simulink model "TosAeb"  (R2021a)
settings: Solver=FixedStepDiscrete  StartTime=0.0  StopTime=inf  FixedStep=0.01  RelTol=1e-3
undefined symbols (not defined in the file or in its folder): ACCModSts, AccSts1, AEB_ObjectMovingOrientation, AEB_ObjectMovingState, CN_BTCActive, CN_CurrentGearR, CN_CurrentGearR_DHT, CN_PTCActive, CN_VDCActive, CN_VSC_EspOff, CN_VSC_FCW_AEB_Error, CN_VSC_FCW_AEB_NoDispay, CN_VSC_FCW_AEB_PerfmDgdtn, CN_VSC_FCW_AEB_TempUnAvlbl, CN_VSC_VehStandstill, CNaccelAvoidMax, CNaccelAvoidMin, CNbrakePedalPress, CNcomfortStrLeftAccelMax, CNcomfortStrLeftAccelMin, CNcomfortStrRightAccelMax, CNcomfortStrRightAccelMin, CNDrvDoorOpen, CNDrvModeEco, CNDrvModeNorm, CNDrvModeOther, CNDrvModeSport, CNDrvSBRreminder, CNNull, CNobjInvalidID, CNPassDoorOpen, CNPassSBRreminder, CNPEBSAccelNegInf, CNPEBSAccelNull, CNPEBSTimeInf, CNPEBSTimeNull, CNSTOPMAX, CNSTOPNULL, CNsubjWidth, CNSwtOFF, CNSwtON, CNTTCMAX, CNTTCNULL, CNVehConfigAEBCycNone, CNVehConfigAEBNone, CNVehConfigAEBPedNone, CNVehConfigFCWNone, CNVehConfigJANone, CollisionSeverity, FALSE, FCWSnvty, JACycObjCalcInfoDefaultParams, JAPedObjCalcInfoDefaultParams, JAVehObjCalcInfoDefaultParams, latCycObjCalcInfoDefaultParams, latPedObjCalcInfoDefaultParams, longObjCalcInfoDefaultParams, ModSts, movingStatus, ObjectType ... (+1112)
libraries: simulink x3031, AEBSFunctionLib x50, Ta_Library x33, AEBSStateMachineLib x23, Mylibrary x12, simulink_extras x7, ObjSelect_lib x3, dspstat3 x2
(part 74 of 102)

== system TosAeb/FcwAebJa_Step_sys/ALG_AEBSystem/ALG_AEBController/AEB_LongTgtInfoCalc/LongObjCalcInfoCalc/AEBS_LongCollisionAssesApp/collisionSeverityHighCalc/collisionSeverityHighCalc2/collisionSeverityHighPhase3/Phase3TimeCalc/tMaxReduceCalc ==
  note: if subjAccelWorkPhase2 < 0
      | 	Calculate tMaxReduce;
      | else
      | 	tMaxReduce = +inf;
  subjLongAccelWorkPhase2 : Inport
  velMaxReudce : Inport  Port=2
  subjVelWorkPhase2 : Inport  Port=3
  If : If  IfExpression=u1 < 0
  Merge : Merge
  TimeMaxReduceCalc : Subsystem  TreatAsAtomicUnit=on
  TimeMaxReduceInf : Subsystem  TreatAsAtomicUnit=on
  tMaxReduce : Outport
  wires:
  If/1 -> TimeMaxReduceCalc/Action
  TimeMaxReduceInf/1(tMaxReduce) -> Merge/2
  TimeMaxReduceCalc/1(tMaxReduce) -> Merge/1
  subjLongAccelWorkPhase2/1 -> If/1, TimeMaxReduceCalc/1(subjLongAccelWorkPhase2)
  velMaxReudce/1 -> TimeMaxReduceCalc/2(velMaxReudce)
  subjVelWorkPhase2/1 -> TimeMaxReduceCalc/3(subjVelWorkPhase2)
  If/2 -> [TIMEINF]
  [TIMEINF] -> TimeMaxReduceInf/Action  "timeMaxReduceInf"
  Merge/1 -> tMaxReduce/1  "tMaxReduce"

== system TosAeb/FcwAebJa_Step_sys/ALG_AEBSystem/ALG_AEBController/AEB_LongTgtInfoCalc/LongObjCalcInfoCalc/AEBS_LongCollisionAssesApp/collisionSeverityHighCalc/collisionSeverityHighCalc2/collisionSeverityHighPhase3/Phase3TimeCalc/tObjStopCalc ==
  accelAbs : Inport
  velAbs : Inport  Port=2
  StopTimeFlow : Stateflow chart  TreatAsAtomicUnit=on
  tStop : Outport
  wires:
  velAbs/1 -> StopTimeFlow/2(velRel)  "velAbs"
  accelAbs/1 -> StopTimeFlow/1(accelRel)  "accelAbs"
  StopTimeFlow/1(tStop) -> tStop/1  "tStop"

== system TosAeb/AEB_Objsel/TA_Processor/AEB_ObjSelect/TargetInfoCheck/Data_precess/TA_EffectCalculate/Obj_FilterFlgCalculate/JA_ObjFilter/CollisionProbCalc/Subsystem/ObjBoundingBox/ObjLLCoordinate ==
  inputSignals : Inport
  Add : Sum  Inputs=+-  OutDataTypeStr=single
  Add1 : Sum  OutDataTypeStr=single
  Bus Selector : BusSelector  OutputSignals=dxPreRefPointObj,ObjWidthCorr,sincosCoef.sinCoef
  Bus Selector1 : BusSelector  OutputSignals=dyPreRefPointObj,ObjWidthCorr,sincosCoef.cosCoef
  Gain : Gain  Gain=0.5  OutDataTypeStr=single
  Gain1 : Gain  Gain=0.5  OutDataTypeStr=single
  Product1 : Product  OutDataTypeStr=single
  Product3 : Product  OutDataTypeStr=single
  dxPre_LLObj : Outport
  dyPreLLObj : Outport  Port=2
  wires:
  Bus Selector1/1 -> Add1/1  "<dyPreRefPointObj>"
  Bus Selector1/3 -> Product3/2  "<cosCoef>"
  Bus Selector1/2 -> Product3/1  "<ObjWidthCorr>"
  Product3/1 -> Gain/1
  Add1/1 -> dyPreLLObj/1
  Add/1 -> dxPre_LLObj/1
  Bus Selector/1 -> Add/1  "<dxPreRefPointObj>"
  Product1/1 -> Gain1/1
  Bus Selector/3 -> Product1/2  "<sinCoef>"
  Bus Selector/2 -> Product1/1  "<ObjWidthCorr>"
  inputSignals/1 -> Bus Selector1/1, Bus Selector/1
  Gain/1 -> Add1/2
  Gain1/1 -> Add/2

== system TosAeb/AEB_Objsel/TA_Processor/AEB_ObjSelect/TargetInfoCheck/Data_precess/TA_EffectCalculate/Obj_FilterFlgCalculate/JA_ObjFilter/CollisionProbCalc/Subsystem/ObjBoundingBox/ObjLUCoordinate ==
  inputSignals : Inport
  Add : Sum  Inputs=++-  OutDataTypeStr=single
  Add1 : Sum  Inputs=+++  OutDataTypeStr=single
  Bus Selector : BusSelector  OutputSignals=dxPreRefPointObj,ObjLengthCorr,sincosCoef.cosCoef,ObjWidthCorr,sincosCoef.sinCoef
  Bus Selector1 : BusSelector  OutputSignals=dyPreRefPointObj,ObjLengthCorr,sincosCoef.sinCoef,ObjWidthCorr,sincosCoef.cosCoef
  Gain : Gain  Gain=0.5  OutDataTypeStr=single
  Gain1 : Gain  Gain=0.5  OutDataTypeStr=single
  Product{,1..3} (4 alike) : Product  OutDataTypeStr=single
  dxPreLUObj : Outport
  dyPreLUobj : Outport  Port=2
  wires:
  inputSignals/1 -> Bus Selector1/1, Bus Selector/1
  Bus Selector/2 -> Product/1  "<ObjLengthCorr>"
  Bus Selector/3 -> Product/2  "<cosCoef>"
  Bus Selector/4 -> Product1/1  "<ObjWidthCorr>"
  Bus Selector/5 -> Product1/2  "<sinCoef>"
  Product1/1 -> Gain1/1
  Bus Selector/1 -> Add/1  "<dxPreRefPointObj>"
  Product/1 -> Add/2
  Add/1 -> dxPreLUObj/1
  Add1/1 -> dyPreLUobj/1
  Product2/1 -> Add1/2
  Product3/1 -> Gain/1
  Bus Selector1/2 -> Product2/1  "<ObjLengthCorr>"
  Bus Selector1/4 -> Product3/1  "<ObjWidthCorr>"
  Bus Selector1/3 -> Product2/2  "<sinCoef>"
  Bus Selector1/5 -> Product3/2  "<cosCoef>"
  Bus Selector1/1 -> Add1/1  "<dyPreRefPointObj>"
  Gain/1 -> Add1/3
  Gain1/1 -> Add/3

== system TosAeb/AEB_Objsel/TA_Processor/AEB_ObjSelect/TargetInfoCheck/Data_precess/TA_EffectCalculate/Obj_FilterFlgCalculate/JA_ObjFilter/CollisionProbCalc/Subsystem/ObjBoundingBox/ObjRLCoordinate ==
  inputSignals : Inport
  Add : Sum  OutDataTypeStr=single
  Add1 : Sum  Inputs=+-  OutDataTypeStr=single
  Bus Selector : BusSelector  OutputSignals=dxPreRefPointObj,ObjWidthCorr,sincosCoef.sinCoef
  Bus Selector1 : BusSelector  OutputSignals=dyPreRefPointObj,ObjWidthCorr,sincosCoef.cosCoef
  Gain : Gain  Gain=0.5  OutDataTypeStr=single
  Gain1 : Gain  Gain=0.5  OutDataTypeStr=single
  Product1 : Product  OutDataTypeStr=single
  Product3 : Product  OutDataTypeStr=single
  dxPreRLObj : Outport
  dyPreRLObj : Outport  Port=2
  wires:
  Bus Selector1/1 -> Add1/1  "<dyPreRefPointObj>"
  Bus Selector1/3 -> Product3/2  "<cosCoef>"
  Bus Selector1/2 -> Product3/1  "<ObjWidthCorr>"
  Product3/1 -> Gain1/1
  Add1/1 -> dyPreRLObj/1
  Add/1 -> dxPreRLObj/1
  Bus Selector/1 -> Add/1  "<dxPreRefPointObj>"
  Product1/1 -> Gain/1
  Bus Selector/3 -> Product1/2  "<sinCoef>"
  Bus Selector/2 -> Product1/1  "<ObjWidthCorr>"
  inputSignals/1 -> Bus Selector1/1, Bus Selector/1
  Gain/1 -> Add/2
  Gain1/1 -> Add1/2

== system TosAeb/AEB_Objsel/TA_Processor/AEB_ObjSelect/TargetInfoCheck/Data_precess/TA_EffectCalculate/Obj_FilterFlgCalculate/JA_ObjFilter/CollisionProbCalc/Subsystem/ObjBoundingBox/ObjRUCoordinate ==
  inputSignals : Inport
  Add : Sum  Inputs=+++  OutDataTypeStr=single
  Add1 : Sum  Inputs=++-  OutDataTypeStr=single
  Bus Selector : BusSelector  OutputSignals=dxPreRefPointObj,ObjLengthCorr,sincosCoef.cosCoef,ObjWidthCorr,sincosCoef.sinCoef
  Bus Selector1 : BusSelector  OutputSignals=dyPreRefPointObj,ObjLengthCorr,sincosCoef.sinCoef,ObjWidthCorr,sincosCoef.cosCoef
  Gain : Gain  Gain=0.5  OutDataTypeStr=single
  Gain1 : Gain  Gain=0.5  OutDataTypeStr=single
  Product{,1..3} (4 alike) : Product  OutDataTypeStr=single
  dxPreRUObj : Outport
  dyPreRUObj : Outport  Port=2
  wires:
  inputSignals/1 -> Bus Selector1/1, Bus Selector/1
  Bus Selector/2 -> Product/1  "<ObjLengthCorr>"
  Bus Selector/3 -> Product/2  "<cosCoef>"
  Bus Selector/4 -> Product1/1  "<ObjWidthCorr>"
  Bus Selector/5 -> Product1/2  "<sinCoef>"
  Product1/1 -> Gain1/1
  Bus Selector/1 -> Add/1  "<dxPreRefPointObj>"
  Product/1 -> Add/2
  Add/1 -> dxPreRUObj/1
  Add1/1 -> dyPreRUObj/1
  Product2/1 -> Add1/2
  Product3/1 -> Gain/1
  Bus Selector1/2 -> Product2/1  "<ObjLengthCorr>"
  Bus Selector1/4 -> Product3/1  "<ObjWidthCorr>"
  Bus Selector1/3 -> Product2/2  "<sinCoef>"
  Bus Selector1/5 -> Product3/2  "<cosCoef>"
  Bus Selector1/1 -> Add1/1  "<dyPreRefPointObj>"
  Gain/1 -> Add1/3
  Gain1/1 -> Add/3

== system TosAeb/AEB_Objsel/TA_Processor/AEB_ObjSelect/TargetInfoCheck/Data_precess/TA_EffectCalculate/Obj_FilterFlgCalculate/JA_ObjFilter/CollisionProbCalc/Subsystem/ObjBoundingBoxRebuild/Subsystem ==
  SubDxFR : Inport
  SubDxFL : Inport  Port=2
  SubDxRL : Inport  Port=3
  SubDxRR : Inport  Port=4
  Add{,1..3} (4 alike) : Sum  Inputs=+-
  Gain : Gain  Gain=1/3
  Gain1 : Gain  Gain=1/3
  Out2 : Outport
  Out1 : Outport  Port=2
  wires:
  SubDxFR/1 -> Add1/1, Add/1
  SubDxRR/1 -> Add/2
  Add/1 -> Gain/1
  Gain/1 -> Add1/2
  Add1/1 -> Out1/1
  Add2/1 -> Gain1/1
  Gain1/1 -> Add3/2
  Add3/1 -> Out2/1
  SubDxRL/1 -> Add2/2
  SubDxFL/1 -> Add3/1, Add2/1

== system TosAeb/AEB_Objsel/TA_Processor/AEB_ObjSelect/TargetInfoCheck/Data_precess/TA_EffectCalculate/Obj_FilterFlgCalculate/JA_ObjFilter/CollisionProbCalc/Subsystem/ObjBoundingBoxRebuild/Subsystem1 ==
  SubDyFR : Inport
  SubDyFL : Inport  Port=2
  SubDyRL : Inport  Port=3
  SubDyRR : Inport  Port=4
  Add{,1..3} (4 alike) : Sum  Inputs=+-
  Gain : Gain  Gain=1/3
  Gain1 : Gain  Gain=1/3
  Out2 : Outport
  Out1 : Outport  Port=2
  wires:
  SubDyFL/1 -> Add2/1, Add3/1
  SubDyRL/1 -> Add2/2
  Add3/1 -> Out2/1
  Gain1/1 -> Add3/2
  Add2/1 -> Gain1/1
  Add1/1 -> Out1/1
  Gain/1 -> Add1/2
  Add/1 -> Gain/1
  SubDyRR/1 -> Add/2
  SubDyFR/1 -> Add/1, Add1/1

== system TosAeb/AEB_Objsel/TA_Processor/AEB_ObjSelect/TargetInfoCheck/Data_precess/TA_EffectCalculate/Obj_FilterFlgCalculate/JA_ObjFilter/CollisionProbCalc/Subsystem/ObjBoundingBoxRebuild/Subsystem2 ==
  ObjDxFR : Inport
  ObjDxFL : Inport  Port=2
  ObjDxRL : Inport  Port=3
  ObjDxRR : Inport  Port=4
  Add : Sum  Inputs=+-
  Add1 : Sum
  Add2 : Sum  Inputs=+-
  Add3 : Sum
  Gain : Gain  Gain=1/3
  Gain1 : Gain  Gain=1/3
  Out2 : Outport
  Out1 : Outport  Port=2
  wires:
  Add/1 -> Gain/1
  Add1/1 -> Out1/1
  Add2/1 -> Gain1/1
  Add3/1 -> Out2/1
  ObjDxRL/1 -> Add3/2, Add2/2
  ObjDxFL/1 -> Add2/1
  ObjDxFR/1 -> Add/1
  ObjDxRR/1 -> Add1/2, Add/2
  Gain/1 -> Add1/1
  Gain1/1 -> Add3/1

== system TosAeb/AEB_Objsel/TA_Processor/AEB_ObjSelect/TargetInfoCheck/Data_precess/TA_EffectCalculate/Obj_FilterFlgCalculate/JA_ObjFilter/CollisionProbCalc/Subsystem/ObjBoundingBoxRebuild/Subsystem3 ==
  ObjDyFR : Inport
  ObjDyFL : Inport  Port=2
  ObjDyRL : Inport  Port=3
  ObjDyRR : Inport  Port=4
  Add : Sum  Inputs=+-
  Add1 : Sum
  Add2 : Sum  Inputs=+-
  Add3 : Sum
  Gain : Gain  Gain=1/3
  Gain1 : Gain  Gain=1/3
  Out2 : Outport
  Out1 : Outport  Port=2
  wires:
  ObjDyRR/1 -> Add1/2, Add/2
  ObjDyFR/1 -> Add/1
  ObjDyFL/1 -> Add2/1
  ObjDyRL/1 -> Add3/2, Add2/2
  Add3/1 -> Out2/1
  Add2/1 -> Gain1/1
  Add1/1 -> Out1/1
  Add/1 -> Gain/1
  Gain1/1 -> Add3/1
  Gain/1 -> Add1/1

== system TosAeb/AEB_Objsel/TA_Processor/AEB_ObjSelect/TargetInfoCheck/Data_precess/TA_EffectCalculate/Obj_FilterFlgCalculate/JA_ObjFilter/CollisionProbCalc/Subsystem/PreCrossFlgCalcFlow/VectorCrossAlgorithm/If Action Subsystem ==
  Action Port : ActionPort  ActionPortLabel=if(u1)
  Constant : Constant  Value=0  OutDataTypeStr=boolean
  CrossFlg : Outport
  wires:
  Constant/1 -> CrossFlg/1

== system TosAeb/AEB_Objsel/TA_Processor/AEB_ObjSelect/TargetInfoCheck/Data_precess/TA_EffectCalculate/Obj_FilterFlgCalculate/JA_ObjFilter/CollisionProbCalc/Subsystem/PreCrossFlgCalcFlow/VectorCrossAlgorithm/If Action Subsystem1 ==
  vectorMulit : Inport
  Coordinations : Inport  Port=2
  Action Port : ActionPort  ActionPortLabel=else
  Bus Selector : BusSelector  OutputSignals=m2,m1,y1,n1,n2,x1
  Bus Selector1 : BusSelector  OutputSignals=x2,x1,y1,n1,y2,m1
  Subsystem : Subsystem
  t : Subsystem
  u : Subsystem
  CrossFlg : Outport
  wires:
  Coordinations/1 -> Bus Selector1/1, Bus Selector/1
  Bus Selector/2 -> t/2(m1)  "<m1>"
  Bus Selector/3 -> t/3(y1)  "<y1>"
  Bus Selector/1 -> t/1(m2)  "<m2>"
  Bus Selector/4 -> t/4(n1)  "<n1>"
  Bus Selector/5 -> t/5(n2)  "<n2>"
  Bus Selector/6 -> t/6(x1)  "<x1>"
  Bus Selector1/3 -> u/3(y1)  "<y1>"
  Bus Selector1/2 -> u/2(x1)  "<x1>"
  Bus Selector1/4 -> u/4(n1)  "<n1>"
  Bus Selector1/1 -> u/1(x2)  "<x2>"
  Bus Selector1/5 -> u/5(y2)  "<y2>"
  Bus Selector1/6 -> u/6(m1)  "<m1>"
  t/1(temp_t) -> Subsystem/1(temp_u)
  u/1(temp_u) -> Subsystem/2(temp_t)
  Subsystem/1(CrossFlg) -> CrossFlg/1
  vectorMulit/1 -> Subsystem/3(vectorMulit)

== system TosAeb/AEB_Objsel/TA_Processor/AEB_ObjSelect/TargetInfoCheck/Data_precess/TA_EffectCalculate/Obj_FilterFlgCalculate/JA_ObjFilter/CollisionProbCalc/Subsystem/PreCrossFlgCalcFlow/VectorCrossAlgorithm/VectorMulit ==
  x1 : Inport  OutDataTypeStr=single
  x2 : Inport  Port=2  OutDataTypeStr=single
  n2 : Inport  Port=3  OutDataTypeStr=single
  n1 : Inport  Port=4  OutDataTypeStr=single
  y2 : Inport  Port=5  OutDataTypeStr=single
  y1 : Inport  Port=6  OutDataTypeStr=single
  m2 : Inport  Port=7  OutDataTypeStr=single
  m1 : Inport  Port=8  OutDataTypeStr=single
  Product : Product  OutDataTypeStr=single
  Product1 : Product  OutDataTypeStr=single
  Subtract{1..4} (4 alike) : Sum  Inputs=+-  OutDataTypeStr=single
  Subtract5 : Sum  Inputs=+-
  vectorMulit : Outport
  wires:
  x1/1 -> Subtract2/2
  x2/1 -> Subtract2/1
  Subtract2/1 -> Product/1
  Subtract3/1 -> Product/2
  y2/1 -> Subtract4/1
  y1/1 -> Subtract4/2
  m2/1 -> Subtract1/1
  m1/1 -> Subtract1/2
  Subtract4/1 -> Product1/1
  Subtract1/1 -> Product1/2
  Product/1 -> Subtract5/1
  Product1/1 -> Subtract5/2
  Subtract5/1 -> vectorMulit/1
  n2/1 -> Subtract3/1
  n1/1 -> Subtract3/2

== system TosAeb/AEB_Objsel/TA_Processor/AEB_ObjSelect/TargetInfoCheck/Data_precess/TA_EffectCalculate/Obj_FilterFlgCalculate/JA_ObjFilter/CollisionProbCalc/Subsystem/SubjBoundingBox/SubjLLCoordinate ==
  inputSignals : Inport
  Add2 : Sum  Inputs=+--  OutDataTypeStr=single
  Add3 : Sum  Inputs=+-+  OutDataTypeStr=single
  Bus Selector2 : BusSelector  OutputSignals=dxPreRefPoint,dxRearBumperToRearAxis,sincosCoef.cosCoef,subjWidth,sincosCoef.sinCoef
  Bus Selector3 : BusSelector  OutputSignals=dyPreRefPoint,dxRearBumperToRearAxis,sincosCoef.sinCoef,subjWidth,sincosCoef.cosCoef
  Gain2 : Gain  Gain=0.5  OutDataTypeStr=single
  Gain3 : Gain  Gain=0.5  OutDataTypeStr=single
  Product{4..7} (4 alike) : Product  OutDataTypeStr=single
  dxPreLL : Outport
  dyPreLL : Outport  Port=2
  wires:
  Gain3/1 -> Add3/3
  Bus Selector3/3 -> Product6/2  "<sinCoef>"
  Add3/1 -> dyPreLL/1
  Product7/1 -> Gain3/1
  Product6/1 -> Add3/2
  Product4/1 -> Add2/2
  Bus Selector3/5 -> Product7/2  "<cosCoef>"
  Product5/1 -> Gain2/1
  Gain2/1 -> Add2/3
  Add2/1 -> dxPreLL/1
  Bus Selector3/4 -> Product7/1  "<subjWidth>"
  Bus Selector3/2 -> Product6/1  "<dxRearBumperToRearAxis>"
  Bus Selector3/1 -> Add3/1  "<dyPreRefPoint>"
  inputSignals/1 -> Bus Selector3/1, Bus Selector2/1
  Bus Selector2/4 -> Product5/1  "<subjWidth>"
  Bus Selector2/2 -> Product4/1  "<dxRearBumperToRearAxis>"
  Bus Selector2/3 -> Product4/2  "<cosCoef>"
  Bus Selector2/1 -> Add2/1  "<dxPreRefPoint>"
  Bus Selector2/5 -> Product5/2  "<sinCoef>"

== system TosAeb/AEB_Objsel/TA_Processor/AEB_ObjSelect/TargetInfoCheck/Data_precess/TA_EffectCalculate/Obj_FilterFlgCalculate/JA_ObjFilter/CollisionProbCalc/Subsystem/SubjBoundingBox/SubjLUCoordinate ==
  inputSignals : Inport
  Add : Sum  Inputs=++-  OutDataTypeStr=single
  Add1 : Sum  Inputs=+++  OutDataTypeStr=single
  Bus Selector : BusSelector  OutputSignals=dxPreRefPoint,dxFrontBumperToRearAxis,sincosCoef.cosCoef,subjWidth,sincosCoef.sinCoef
  Bus Selector1 : BusSelector  OutputSignals=dyPreRefPoint,dxFrontBumperToRearAxis,sincosCoef.sinCoef,subjWidth,sincosCoef.cosCoef
  Gain : Gain  Gain=0.5  OutDataTypeStr=single
  Gain1 : Gain  Gain=0.5  OutDataTypeStr=single
  Product{,1..3} (4 alike) : Product  OutDataTypeStr=single
  dxPreLU : Outport
  dyPreLU : Outport  Port=2
  wires:
  Bus Selector/4 -> Product1/1  "<subjWidth>"
  Product3/1 -> Gain1/1
  Add/1 -> dxPreLU/1
  Bus Selector1/1 -> Add1/1  "<dyPreRefPoint>"
  Gain1/1 -> Add1/3
  Bus Selector1/4 -> Product3/1  "<subjWidth>"
  Product2/1 -> Add1/2
  Bus Selector/3 -> Product/2  "<cosCoef>"
  Add1/1 -> dyPreLU/1
  Bus Selector/5 -> Product1/2  "<sinCoef>"
  Bus Selector/2 -> Product/1  "<dxFrontBumperToRearAxis>"
  Bus Selector1/2 -> Product2/1  "<dxFrontBumperToRearAxis>"
  inputSignals/1 -> Bus Selector1/1, Bus Selector/1
  Bus Selector1/5 -> Product3/2  "<cosCoef>"
  Bus Selector/1 -> Add/1  "<dxPreRefPoint>"
  Gain/1 -> Add/3
  Product1/1 -> Gain/1
  Product/1 -> Add/2
  Bus Selector1/3 -> Product2/2  "<sinCoef>"

== system TosAeb/AEB_Objsel/TA_Processor/AEB_ObjSelect/TargetInfoCheck/Data_precess/TA_EffectCalculate/Obj_FilterFlgCalculate/JA_ObjFilter/CollisionProbCalc/Subsystem/SubjBoundingBox/SubjRLCoordinate ==
  inputSignals : Inport
  Add6 : Sum  Inputs=+-+  OutDataTypeStr=single
  Add7 : Sum  Inputs=+--  OutDataTypeStr=single
  Bus Selector6 : BusSelector  OutputSignals=dxPreRefPoint,dxRearBumperToRearAxis,sincosCoef.cosCoef,subjWidth,sincosCoef.sinCoef
  Bus Selector7 : BusSelector  OutputSignals=dyPreRefPoint,dxRearBumperToRearAxis,sincosCoef.sinCoef,subjWidth,sincosCoef.cosCoef
  Gain6 : Gain  Gain=0.5  OutDataTypeStr=single
  Gain7 : Gain  Gain=0.5  OutDataTypeStr=single
  Product{12..15} (4 alike) : Product  OutDataTypeStr=single
  dxPreRL : Outport
  dyPreRL : Outport  Port=2
  wires:
  Product12/1 -> Add6/2
  Gain6/1 -> Add6/3
  Product13/1 -> Gain6/1
  Add6/1 -> dxPreRL/1
  Bus Selector6/4 -> Product13/1  "<subjWidth>"
  Bus Selector6/5 -> Product13/2  "<sinCoef>"
  Bus Selector6/1 -> Add6/1  "<dxPreRefPoint>"
  Bus Selector6/2 -> Product12/1  "<dxRearBumperToRearAxis>"
  Bus Selector6/3 -> Product12/2  "<cosCoef>"
  inputSignals/1 -> Bus Selector7/1, Bus Selector6/1
  Add7/1 -> dyPreRL/1
  Product14/1 -> Add7/2
  Gain7/1 -> Add7/3
  Bus Selector7/1 -> Add7/1  "<dyPreRefPoint>"
  Bus Selector7/2 -> Product14/1  "<dxRearBumperToRearAxis>"
  Bus Selector7/4 -> Product15/1  "<subjWidth>"
  Product15/1 -> Gain7/1
  Bus Selector7/5 -> Product15/2  "<cosCoef>"
  Bus Selector7/3 -> Product14/2  "<sinCoef>"

== system TosAeb/AEB_Objsel/TA_Processor/AEB_ObjSelect/TargetInfoCheck/Data_precess/TA_EffectCalculate/Obj_FilterFlgCalculate/JA_ObjFilter/CollisionProbCalc/Subsystem/SubjBoundingBox/SubjRUCoordinate ==
  inputSignals : Inport
  Add4 : Sum  Inputs=+++  OutDataTypeStr=single
  Add5 : Sum  Inputs=++-  OutDataTypeStr=single
  Bus Selector4 : BusSelector  OutputSignals=dxPreRefPoint,dxFrontBumperToRearAxis,sincosCoef.cosCoef,subjWidth,sincosCoef.sinCoef
  Bus Selector5 : BusSelector  OutputSignals=dyPreRefPoint,dxFrontBumperToRearAxis,sincosCoef.sinCoef,subjWidth,sincosCoef.cosCoef
  Gain4 : Gain  Gain=0.5  OutDataTypeStr=single
  Gain5 : Gain  Gain=0.5  OutDataTypeStr=single
  Product{8..11} (4 alike) : Product  OutDataTypeStr=single
  dxPreRU : Outport
  dyPreRU : Outport  Port=2
  wires:
  Bus Selector4/4 -> Product9/1  "<subjWidth>"
  Bus Selector4/5 -> Product9/2  "<sinCoef>"
  Bus Selector4/3 -> Product8/2  "<cosCoef>"
  Bus Selector4/1 -> Add4/1  "<dxPreRefPoint>"
  inputSignals/1 -> Bus Selector5/1, Bus Selector4/1
  Bus Selector4/2 -> Product8/1  "<dxFrontBumperToRearAxis>"
  Product9/1 -> Gain4/1
  Bus Selector5/4 -> Product11/1  "<subjWidth>"
  Product10/1 -> Add5/2
  Product11/1 -> Gain5/1
  Add5/1 -> dyPreRU/1
  Gain5/1 -> Add5/3
  Bus Selector5/2 -> Product10/1  "<dxFrontBumperToRearAxis>"
  Bus Selector5/3 -> Product10/2  "<sinCoef>"
  Bus Selector5/5 -> Product11/2  "<cosCoef>"
  Add4/1 -> dxPreRU/1
  Gain4/1 -> Add4/3
  Product8/1 -> Add4/2
  Bus Selector5/1 -> Add5/1  "<dyPreRefPoint>"

== system TosAeb/AEB_Objsel/TA_Processor/AEB_ObjSelect/TargetInfoCheck/Data_precess/TA_EffectCalculate/Obj_FilterFlgCalculate/JA_ObjFilter/CollisionProbCalc/Subsystem/Subsystem/CalculateHeading ==
  dx : Inport
  dy : Inport  Port=2
  atan2 : Trigonometry  Operator=atan2
  PredHostHeading : Outport
  wires:
  dx/1 -> atan2/1
  dy/1 -> atan2/2
  atan2/1 -> PredHostHeading/1  "PredHostHeading"

== system TosAeb/AEB_Objsel/TA_Processor/AEB_ObjSelect/TargetInfoCheck/Data_precess/TA_EffectCalculate/Obj_FilterFlgCalculate/JA_ObjFilter/CollisionProbCalc/Subsystem/Subsystem/SegmentComponents ==
  note: 判断预测时间的位置，与第一段时间做差，然后除以第一段与第四段的差值，输出数
      | 值为0到1的数
  note: 驾驶员预测前方道路分为4段，输出为每段的4个点的横纵坐标值，然后做拟合处理
  PahEstimn : Inport
  PredictionTime : Inport  Port=2
  Add : Sum
  Bus Selector : BusSelector  OutputSignals=CtrlAryLgt,CtrlAryLat
  Compare To Constant : simulink/Logic and Bit Operations/Compare To Constant  relop=<=  const=1  OutDataTypeStr=boolean
  Constant : Constant  OutDataTypeStr=single
  LatPosition : MATLAB Function  TreatAsAtomicUnit=on
  LongPosition : MATLAB Function  TreatAsAtomicUnit=on
  Selector : Selector  InputPortWidth=4  IndexOptions=Index vector (dialog)  Indices=1  OutputSizes=1
  Selector1 : Selector  InputPortWidth=4  IndexOptions=Index vector (dialog)  Indices=2  OutputSizes=1
  Selector2 : Selector  InputPortWidth=4  IndexOptions=Index vector (dialog)  Indices=3  OutputSizes=1
  Selector3 : Selector  InputPortWidth=4  IndexOptions=Index vector (dialog)  Indices=4  OutputSizes=1
  Selector4 : Selector  InputPortWidth=4  IndexOptions=Index vector (dialog)  Indices=1  OutputSizes=1
  Selector5 : Selector  InputPortWidth=4  IndexOptions=Index vector (dialog)  Indices=2  OutputSizes=1
  Selector6 : Selector  InputPortWidth=4  IndexOptions=Index vector (dialog)  Indices=3  OutputSizes=1
  Selector7 : Selector  InputPortWidth=4  IndexOptions=Index vector (dialog)  Indices=4  OutputSizes=1
  Switch : Switch  Criteria=u2 ~= 0
  PredHostPosnLgt : Outport
  dx : Outport  Port=2
  PredHostPosnLat : Outport  Port=3
  dy : Outport  Port=4
  wires:
  Bus Selector/2 -> Selector6/1, Selector7/1, Selector4/1, Selector5/1  "<CtrlAryLat>"
  Bus Selector/1 -> Selector2/1, Selector1/1, Selector/1, Selector3/1  "<CtrlAryLgt>"
  PahEstimn/1 -> Bus Selector/1
  Selector1/1 -> LongPosition/2(P1)  "P1x"
  Selector/1 -> LongPosition/1(P0)  "P0x"
  Selector2/1 -> LongPosition/3(P2)  "P2x"
  Selector3/1 -> LongPosition/4(P3)  "P3x"
  Selector4/1 -> LatPosition/1(P0)  "P0y"
  Selector5/1 -> LatPosition/2(P1)  "P1y"
  Selector6/1 -> LatPosition/3(P2)  "P2y"
  Selector7/1 -> LatPosition/4(P3)  "P3y"
  LongPosition/1(x) -> PredHostPosnLgt/1  "PredHostPosnLgt"
  LongPosition/2(dx) -> dx/1
  LatPosition/1(y) -> PredHostPosnLat/1  "PredHostPosnLat"
  LatPosition/2(dy) -> dy/1
  PredictionTime/1 -> Compare To Constant/1, Add/1, Switch/1
  Compare To Constant/1 -> Switch/2
  Constant/1 -> Add/2
  Add/1 -> Switch/3
  Switch/1 -> [tau]
  [tau] -> LatPosition/5(tau)
  [tau] -> LongPosition/5(tau)

== system TosAeb/AEB_Objsel/TA_Processor/AEB_ObjSelect/TargetInfoCheck/Data_precess/TA_EffectCalculate/Obj_FilterFlgCalculate/JA_ObjFilter/CollisionProbCalc/Subsystem/ay_Filt/ay1 ==
  ay : Inport
  Bus Creator : BusCreator  Inputs=2
  Bus Creator1 : BusCreator  Inputs=2
  Compare To Constant : simulink/Logic and Bit Operations/Compare To Constant  relop=>=  const=0.01  OutDataTypeStr=boolean
  Constant : Constant  Value=0  OutDataTypeStr=single
  Constant1 : Constant  Value=0  OutDataTypeStr=single
  Constant2 : Constant  OutDataTypeStr=single
  Switch : Switch  Criteria=u2 ~= 0
  Out_ay1 : Outport
  wires:
  Compare To Constant/1 -> Switch/2
  Constant/1 -> Bus Creator/1  "Count1"
  Constant1/1 -> Bus Creator/2  "ay1"
  ay/1 -> Bus Creator1/2, Compare To Constant/1  "ay1"
  Bus Creator1/1 -> Switch/1
  Switch/1 -> Out_ay1/1  "Out_ay1"
  Bus Creator/1 -> Switch/3
  Constant2/1 -> Bus Creator1/1  "Count1"

== system TosAeb/AEB_Objsel/TA_Processor/AEB_ObjSelect/TargetInfoCheck/Data_precess/TA_EffectCalculate/Obj_FilterFlgCalculate/JA_ObjFilter/CollisionProbCalc/Subsystem/ay_Filt/ay2 ==
  ay : Inport
  Bus Creator : BusCreator  Inputs=2
  Bus Creator1 : BusCreator  Inputs=2
  Compare To Constant : simulink/Logic and Bit Operations/Compare To Constant  relop=>=  const=0.01  OutDataTypeStr=boolean
  Constant : Constant  Value=0  OutDataTypeStr=single
  Constant1 : Constant  Value=0  OutDataTypeStr=single
  Constant2 : Constant  OutDataTypeStr=single
  Switch : Switch  Criteria=u2 > Threshold
  Out_ay2 : Outport
  wires:
  Constant2/1 -> Bus Creator1/1  "Count2"
  Bus Creator/1 -> Switch/3
  Switch/1 -> Out_ay2/1  "Out_ay2"
  Bus Creator1/1 -> Switch/1
  ay/1 -> Compare To Constant/1, Bus Creator1/2  "ay2"
  Constant1/1 -> Bus Creator/2  "ay2"
  Constant/1 -> Bus Creator/1  "Count2"
  Compare To Constant/1 -> Switch/2

== system TosAeb/AEB_Objsel/TA_Processor/AEB_ObjSelect/TargetInfoCheck/Data_precess/TA_EffectCalculate/Obj_FilterFlgCalculate/JA_ObjFilter/CollisionProbCalc/Subsystem/ay_Filt/ay3 ==
  ay : Inport
  Bus Creator : BusCreator  Inputs=2
  Bus Creator1 : BusCreator  Inputs=2
  Compare To Constant : simulink/Logic and Bit Operations/Compare To Constant  relop=>=  const=0.01  OutDataTypeStr=boolean
  Constant : Constant  Value=0  OutDataTypeStr=single
  Constant1 : Constant  Value=0  OutDataTypeStr=single
  Constant2 : Constant  OutDataTypeStr=single
  Switch : Switch  Criteria=u2 > Threshold
  Out_ay3 : Outport
  wires:
  Compare To Constant/1 -> Switch/2
  Constant/1 -> Bus Creator/1  "Count3"
  Constant1/1 -> Bus Creator/2  "ay3"
  ay/1 -> Bus Creator1/2, Compare To Constant/1  "ay3"
  Bus Creator1/1 -> Switch/1
  Switch/1 -> Out_ay3/1  "Out_ay3"
  Bus Creator/1 -> Switch/3
  Constant2/1 -> Bus Creator1/1  "Count3"

== system TosAeb/AEB_Objsel/TA_Processor/AEB_ObjSelect/TargetInfoCheck/Data_precess/TA_EffectCalculate/Obj_FilterFlgCalculate/JA_ObjFilter/CollisionProbCalc/Subsystem/ay_Filt/ay4 ==
  ay : Inport
  Bus Creator : BusCreator  Inputs=2
  Bus Creator1 : BusCreator  Inputs=2
  Compare To Constant : simulink/Logic and Bit Operations/Compare To Constant  relop=>=  const=0.01  OutDataTypeStr=boolean
  Constant : Constant  Value=0  OutDataTypeStr=single
  Constant1 : Constant  Value=0  OutDataTypeStr=single
  Constant2 : Constant  OutDataTypeStr=single
  Switch : Switch  Criteria=u2 > Threshold
  Out_ay4 : Outport
  wires:
  Constant2/1 -> Bus Creator1/1  "Count4"
  Bus Creator/1 -> Switch/3
  Switch/1 -> Out_ay4/1  "Out_ay4"
  Bus Creator1/1 -> Switch/1
  ay/1 -> Compare To Constant/1, Bus Creator1/2  "ay4"
  Constant1/1 -> Bus Creator/2  "ay4"
  Constant/1 -> Bus Creator/1  "Count4"
  Compare To Constant/1 -> Switch/2

== system TosAeb/AEB_Objsel/TA_Processor/AEB_ObjSelect/TargetInfoCheck/Data_precess/TA_EffectCalculate/Obj_FilterFlgCalculate/Lat_ObjFilter/Lat_Objelse/Lat_ObjPre/Subsystem/LatDis_Cal ==
  note: 自车与目标当前状态下无重叠
  dy : Inport
  Width : Inport  Port=2
  Oreintation : Inport  Port=3
  vy : Inport  Port=4
  dx : Inport  Port=5
  ax : Inport  Port=6
  ay : Inport  Port=7
  vx : Inport  Port=8
  If : If  NumInputs=2  ElseIfExpressions=u2 > 0
  L2R : Subsystem
  Merge : Merge  Inputs=3
  Merge1 : Merge  Inputs=3
  Obj_PosnJudge : Subsystem
  R2L : Subsystem
  Width_Calculate : Subsystem
  others : Subsystem
  Lat_ObjIntersect : Outport
  Lat_TTR : Outport  Port=2
  wires:
  Oreintation/1 -> Obj_PosnJudge/1(Oreintation)
  vy/1 -> [vy], Obj_PosnJudge/2(vy)
  Obj_PosnJudge/1(L2R) -> If/1
  Obj_PosnJudge/2(R2L) -> If/2
  dy/1 -> [dy], L2R/1(dy)
  Width/1 -> Width_Calculate/1(Width)
  Width_Calculate/1(Width_Large) -> L2R/2(Width_Large), [Width_Large]
  Width_Calculate/2(Width_half) -> [Width_half], L2R/3(Width_half)
  dx/1 -> [dx], L2R/4(dx)
  ax/1 -> [ax], L2R/5(ax)
  vx/1 -> [vx], L2R/7(vx)
  ay/1 -> [ay], L2R/6(ay)
  [vy] -> L2R/8(vy)
  If/1 -> L2R/Action
  If/2 -> R2L/Action
  [vy] -> R2L/8(vy)
  [dy] -> R2L/1(dy)
  [Width_Large] -> R2L/2(Width_Large)
  [Width_half] -> R2L/3(Width_half)
  [dx] -> R2L/4(dx)
  [ay] -> R2L/6(ay)
  [ax] -> R2L/5(ax)
  [vx] -> R2L/7(vx)
  If/3 -> others/Action
  L2R/1(Valid_Flg) -> Merge/1
  Merge/1 -> Lat_ObjIntersect/1
  R2L/1(Valid_Flg) -> Merge/2
  others/1(Valid_Flg) -> Merge/3
  L2R/2(TTI) -> Merge1/1
  R2L/2(TTI) -> Merge1/2
  others/2(TTI) -> Merge1/3
  Merge1/1 -> Lat_TTR/1

== system TosAeb/AEB_Objsel/TA_Processor/AEB_ObjSelect/TargetInfoCheck/Data_precess/TA_EffectCalculate/Obj_FilterFlgCalculate/Lat_ObjFilter/Lat_Objelse/Lat_ObjPre/Subsystem/LatDis_Cal1 ==
  note: 自车与目标当前状态下无重叠
  dy : Inport
  Width : Inport  Port=2
  Oreintation : Inport  Port=3
  vy : Inport  Port=4
  dx : Inport  Port=5
  ax : Inport  Port=6
  ay : Inport  Port=7
  vx : Inport  Port=8
  If : If  NumInputs=2  ElseIfExpressions=u2 > 0
  L2R : Subsystem
  Merge : Merge  Inputs=3
  Merge1 : Merge  Inputs=3
  Obj_PosnJudge : Subsystem
  R2L : Subsystem
  Width_Calculate : Subsystem
  others : Subsystem
  Lat_ObjIntersect : Outport
  Lat_TTR : Outport  Port=2
  wires:
  Merge1/1 -> Lat_TTR/1
  others/2(TTI) -> Merge1/3
  R2L/2(TTI) -> Merge1/2
  L2R/2(TTI) -> Merge1/1
  others/1(Valid_Flg) -> Merge/3
  R2L/1(Valid_Flg) -> Merge/2
  Merge/1 -> Lat_ObjIntersect/1
  L2R/1(Valid_Flg) -> Merge/1
  If/3 -> others/Action
  [vx] -> R2L/7(vx)
  [ax] -> R2L/5(ax)
  [ay] -> R2L/6(ay)
  [dx] -> R2L/4(dx)
  [Width_half] -> R2L/3(Width_half)
  [Width_Large] -> R2L/2(Width_Large)
  [dy] -> R2L/1(dy)
  [vy] -> R2L/8(vy)
  If/2 -> R2L/Action
  If/1 -> L2R/Action
  [vy] -> L2R/8(vy)
  ay/1 -> L2R/6(ay), [ay]
  vx/1 -> L2R/7(vx), [vx]
  ax/1 -> L2R/5(ax), [ax]
  dx/1 -> L2R/4(dx), [dx]
  Width_Calculate/2(Width_half) -> L2R/3(Width_half), [Width_half]
  Width_Calculate/1(Width_Large) -> [Width_Large], L2R/2(Width_Large)
  Width/1 -> Width_Calculate/1(Width)
  dy/1 -> L2R/1(dy), [dy]
  Obj_PosnJudge/2(R2L) -> If/2
  Obj_PosnJudge/1(L2R) -> If/1
  vy/1 -> Obj_PosnJudge/2(vy), [vy]
  Oreintation/1 -> Obj_PosnJudge/1(Oreintation)

== system TosAeb/AEB_Objsel/TA_Processor/AEB_ObjSelect/TargetInfoCheck/Data_precess/TA_EffectCalculate/Obj_FilterFlgCalculate/Long_ObjFilter/OverlapCalculate/BoudingBoxCalculate_Else/Overlap_FlgCalculate/AbsPosn_InsectionFlg ==
  PosnLgtHost : Inport
  PosnLatHost : Inport  Port=2
  HeadingHost : Inport  Port=3
  Obj_LongPosn : Inport  Port=4
  Obj_LatPosn : Inport  Port=5
  Obj_PreHeading : Inport  Port=6
  Obj_Length : Inport  Port=7
  Obj_Width : Inport  Port=8
  ObjBoundingBox : Subsystem
  Overlap_Judgment : Subsystem
  SubjBoundingBox : Subsystem
  sincosCoefCalc : Subsystem
  sincosCoefCalc1 : Subsystem
  Abs_OverlapFlg : Outport
  wires:
  sincosCoefCalc/1(sincosCoef) -> SubjBoundingBox/3(sincosCoef)
  PosnLatHost/1 -> SubjBoundingBox/2(dyPreRefPoint)
  PosnLgtHost/1 -> SubjBoundingBox/1(dxPreRefPoint)
  sincosCoefCalc1/1(sincosCoef) -> ObjBoundingBox/5(sincosCoef)
  HeadingHost/1 -> sincosCoefCalc/1(angle)
  Obj_Length/1 -> ObjBoundingBox/1(ObjLength)
  Obj_Width/1 -> ObjBoundingBox/2(ObjWidth)
  Obj_LongPosn/1 -> ObjBoundingBox/3(dxPreRefPointObj)
  Obj_LatPosn/1 -> ObjBoundingBox/4(dyPreRefPointObj)
  Obj_PreHeading/1 -> sincosCoefCalc1/1(angle)
  SubjBoundingBox/1(subjDxPre) -> Overlap_Judgment/1(subjDxPre)
  SubjBoundingBox/2(subjDyPre) -> Overlap_Judgment/2(subjDyPre)
  ObjBoundingBox/2(objDyPre) -> Overlap_Judgment/4(objDyPre)
  ObjBoundingBox/1(objDxPre) -> Overlap_Judgment/3(objDxPre)
  Overlap_Judgment/1(Abs_OverlapFlg) -> Abs_OverlapFlg/1

== system TosAeb/AEB_Objsel/TA_Processor/AEB_ObjSelect/TargetInfoCheck/Data_precess/TA_EffectCalculate/Obj_FilterFlgCalculate/Long_ObjFilter/OverlapCalculate/BoudingBoxCalculate_Else/Overlap_FlgCalculate/OverlapFlagJudgement ==
  note: P_TA_PrmForOverlapCalSelect == 1 Overlap 计算采用绝对和相对计算关系
      | P_TA_PrmForOverlapCalSelect == 2 Overlap 计算采用绝对信息计算
      | P_TA_PrmForOverlapCalSelect 等于其他值 Overlap采用相对计算方式
  Abs_OverlapFlg : Inport
  Relative_OverlapFlg : Inport  Port=2
  Compare To Constant : simulink/Logic and Bit Operations/Compare To Constant  relop===  const=1  OutDataTypeStr=boolean
  Compare To Constant1 : simulink/Logic and Bit Operations/Compare To Constant  relop===  const=2  OutDataTypeStr=boolean
  Constant : Constant  Value=P_TA_PrmForOverlapCalSelect
  Constant1 : Constant  Value=P_TA_PrmForOverlapCalSelect
  Constant2 : Constant  OutDataTypeStr=boolean
  Logical Operator : Logic  AllPortsSameDT=off  OutDataTypeStr=boolean
  Switch : Switch  Criteria=u2 ~= 0
  Switch1 : Switch  Criteria=u2 ~= 0
  Terminator : Terminator
  Boudingbox_Calculate : Outport
  wires:
  Abs_OverlapFlg/1 -> Logical Operator/1, Switch1/1
  Relative_OverlapFlg/1 -> Logical Operator/2, Switch1/3
  Constant/1 -> Compare To Constant/1
  Compare To Constant/1 -> Switch/2
  Logical Operator/1 -> Switch/1
  Switch1/1 -> Switch/3
  Constant1/1 -> Compare To Constant1/1
  Compare To Constant1/1 -> Switch1/2
  Constant2/1 -> Boudingbox_Calculate/1
  Switch/1 -> Terminator/1

== system TosAeb/AEB_Objsel/TA_Processor/AEB_ObjSelect/TargetInfoCheck/Data_precess/TA_EffectCalculate/Obj_FilterFlgCalculate/Long_ObjFilter/OverlapCalculate/BoudingBoxCalculate_Else/Overlap_FlgCalculate/RelativePosn_InsectionFlg ==
  Relative_LongPosn : Inport
  Relative_LatPosn : Inport  Port=2
  AEB_State : Inport  Port=3
  Obj_Length : Inport  Port=4
  Obj_Width : Inport  Port=5
  Obj_LatPosn : Inport  Port=6
  PosnLatHost : Inport  Port=7
  Abs : Abs
  Add : Sum  Inputs=+-
  Add1 : Sum
  Compare To Constant : simulink/Logic and Bit Operations/Compare To Constant  relop=~=  const=0  OutDataTypeStr=boolean
  Compare To Constant1 : simulink/Logic and Bit Operations/Compare To Constant  relop=<=  const=P_TA_PrmForOverlapLongThrd  OutDataTypeStr=boolean
  Constant : Constant  Value=0.5
  Constant2 : Constant  Value=0.5  OutDataTypeStr=single
  Constant3 : Constant  Value=P_TA_PrmForVehWidth
  Logical Operator : Logic  AllPortsSameDT=off  OutDataTypeStr=boolean
  Logical Operator1 : Logic  AllPortsSameDT=off  OutDataTypeStr=boolean
  Logical Operator2 : Logic  Operator=NOT  AllPortsSameDT=off  OutDataTypeStr=boolean
  Product1 : Product
  Product2 : Product
  Relational Operator : RelationalOperator  Operator=<=  OutDataTypeStr=boolean  RndMeth=Simplest
  Switch : Switch  Criteria=u2 ~= 0
  Terminator{,1..3} (4 alike) : Terminator
  Relative_OverlapFlg : Outport
  wires:
  Relative_LongPosn/1 -> Compare To Constant1/1
  Constant/1 -> Product1/1
  Obj_Width/1 -> Product1/2
  Constant2/1 -> Product2/1
  Product2/1 -> Add1/2
  Constant3/1 -> Product2/2
  Compare To Constant1/1 -> Logical Operator/1
  Relational Operator/1 -> Logical Operator/2, Logical Operator1/1
  Logical Operator/1 -> Switch/1
  Obj_Length/1 -> Terminator/1
  Product1/1 -> Add1/1
  AEB_State/1 -> Compare To Constant/1
  Compare To Constant/1 -> Logical Operator2/1, Logical Operator1/2
  Logical Operator1/1 -> Switch/3
  Switch/1 -> Relative_OverlapFlg/1
  Logical Operator2/1 -> Switch/2
  Abs/1 -> Relational Operator/1
  Add1/1 -> Relational Operator/2
  Obj_LatPosn/1 -> Add/1, Terminator1/1
  PosnLatHost/1 -> Add/2, Terminator2/1
  Add/1 -> Abs/1
  Relative_LatPosn/1 -> Terminator3/1

== system TosAeb/AEB_Objsel/TA_Processor/AEB_ObjSelect/TargetInfoCheck/Data_precess/TA_EffectCalculate/Obj_FilterFlgCalculate/Long_ObjFilter/OverlapCalculate/BoudingBoxCalculate_Else/PosnatTTI/LongitudinalMotionData ==
  TA_ObjInfo : Inport
  Bus Selector : BusSelector  OutputSignals=TA_ObsVxAbs,TA_ObsVyAbs,TA_ObsAxAbs,TA_ObsAyAbs,TA_ObsDx,TA_ObsDy,TA_ObsVx,TA_ObsVy,TA_ObsAx,TA_ObsAy
  Subsystem1 : Subsystem  Commented=on
  Obj_Vx : Outport
  Obj_Vy : Outport  Port=2
  Obj_Ax : Outport  Port=3
  Obj_Ay : Outport  Port=4
  Obj_dx : Outport  Port=5
  Obj_dy : Outport  Port=6
  Ref_Vx : Outport  Port=7
  Ref_Vy : Outport  Port=8
  Ref_ax : Outport  Port=9
  Ref_ay : Outport  Port=10
  wires:
  TA_ObjInfo/1 -> Bus Selector/1
  Bus Selector/1 -> Obj_Vx/1  "<TA_ObsVxAbs>"
  Bus Selector/2 -> Obj_Vy/1  "<TA_ObsVyAbs>"
  Bus Selector/3 -> Obj_Ax/1  "<TA_ObsAxAbs>"
  Bus Selector/4 -> Obj_Ay/1  "<TA_ObsAyAbs>"
  Bus Selector/5 -> Obj_dx/1  "<TA_ObsDx>"
  Bus Selector/6 -> Obj_dy/1  "<TA_ObsDy>"
  Bus Selector/7 -> Ref_Vx/1  "<TA_ObsVx>"
  Bus Selector/8 -> Ref_Vy/1  "<TA_ObsVy>"
  Bus Selector/9 -> Ref_ax/1  "<TA_ObsAx>"
  Bus Selector/10 -> Ref_ay/1  "<TA_ObsAy>"

== system TosAeb/AEB_Objsel/TA_Processor/AEB_ObjSelect/TargetInfoCheck/Data_precess/TA_EffectCalculate/Obj_FilterFlgCalculate/Long_ObjFilter/OverlapCalculate/BoudingBoxCalculate_Else/PosnatTTI/PredictHostPositionAtTTR ==
  PredictionTime : Inport  PortDimensions=1
  PahEstimn : Inport  Port=2  OutDataTypeStr=Bus: PahEstimn
  VehSelfData : Inport  Port=3
  Bus Selector : BusSelector  OutputSignals=CtrlAryLgt
  Bus Selector1 : BusSelector  OutputSignals=CtrlAryLat
  CalculateHeading : Subsystem  Commented=on
  Constant : Constant  OutDataTypeStr=single
  Constant1 : Constant  OutDataTypeStr=single
  Delay : Delay  DelayLength=1000
  Delay1 : Delay  DelayLength=1000
  HeadingCalculate : Subsystem
  LatPosition : MATLAB Function  TreatAsAtomicUnit=on
  LatPosition1 : MATLAB Function  TreatAsAtomicUnit=on
  LongPosition : MATLAB Function  TreatAsAtomicUnit=on
  LongPosition1 : MATLAB Function  TreatAsAtomicUnit=on
  SegmentComponents : Subsystem
  SelectSegment : Subsystem
  Selector1 : Selector  InputPortWidth=4  IndexOptions=Index vector (dialog)  Indices=3  OutputSizes=1
  Selector2 : Selector  InputPortWidth=4  IndexOptions=Index vector (dialog)  Indices=2  OutputSizes=1
  Selector3 : Selector  InputPortWidth=4  IndexOptions=Index vector (dialog)  Indices=4  OutputSizes=1
  Selector4 : Selector  InputPortWidth=4  IndexOptions=Index vector (dialog)  Indices=1  OutputSizes=1
  Selector5 : Selector  InputPortWidth=4  IndexOptions=Index vector (dialog)  Indices=3  OutputSizes=1
  Selector6 : Selector  InputPortWidth=4  IndexOptions=Index vector (dialog)  Indices=2  OutputSizes=1
  Selector7 : Selector  InputPortWidth=4  IndexOptions=Index vector (dialog)  Indices=4  OutputSizes=1
  Selector8 : Selector  InputPortWidth=4  IndexOptions=Index vector (dialog)  Indices=1  OutputSizes=1
  Terminator : Terminator
  Terminator1 : Terminator
  Terminator_1 : Terminator
  Terminator_2 : Terminator
  PredHostPosnLgt : Outport
  PredHostPosnLat : Outport  Port=2
  PredHostHeading : Outport  Port=3
  wires:
  [tau] -> LongPosition/5(tau)
  LatPosition/1(y) -> PredHostPosnLat/1  "PredHostPosnLat"
  LongPosition/1(x) -> PredHostPosnLgt/1  "PredHostPosnLgt"
  SelectSegment/1(Segment) -> SegmentComponents/1(Segment)
  SegmentComponents/9(tau) -> [tau], LatPosition/5(tau)
  SegmentComponents/8(P3y) -> LatPosition/4(P3)
  SegmentComponents/7(P2y) -> LatPosition/3(P2)
  SegmentComponents/6(P1y) -> LatPosition/2(P1)
  SegmentComponents/5(P0y) -> LatPosition/1(P0)
  SegmentComponents/4(P3x) -> LongPosition/4(P3)
  SegmentComponents/3(P2x) -> LongPosition/3(P2)
  SegmentComponents/2(P1x) -> LongPosition/2(P1)
  SegmentComponents/1(P0x) -> LongPosition/1(P0)
  PahEstimn/1 -> CalculateHeading/1(PathEstimn), SelectSegment/1(PahEstimn)  "PahEstimn"
  PredictionTime/1 -> HeadingCalculate/1(PredictionTime), SegmentComponents/2(PredictionTime), SelectSegment/2(Time)  "PredictionTime"
  LongPosition/2(dx) -> [dx]
  LatPosition/2(dy) -> [dy]
  SelectSegment/2(SegmentIndex) -> [SegmentIndex]
  [SegmentIndex] -> CalculateHeading/2(SegmentIndex)
  [dx] -> CalculateHeading/3(dx)
  [dy] -> CalculateHeading/4(dy)
  VehSelfData/1 -> HeadingCalculate/2(VehSelfData)
  HeadingCalculate/2(Heanding) -> PredHostHeading/1
  HeadingCalculate/1(Curvatrue) -> SegmentComponents/3(Curvatrue)
  SelectSegment/3(Segment1) -> [Segment1]
  [Segment1] -> Bus Selector1/1, Bus Selector/1
  Bus Selector/1 -> Selector2/1, Selector3/1, Selector1/1, Selector4/1  "<CtrlAryLgt>"
  Selector1/1 -> LongPosition1/3(P2)  "P2x"
  Selector3/1 -> LongPosition1/4(P3)  "P3x"
  Selector2/1 -> LongPosition1/2(P1)  "P1x"
  Selector4/1 -> LongPosition1/1(P0)  "P0x"
  Constant/1 -> LongPosition1/5(tau)
  LongPosition1/1(x) -> Delay/1
  Selector6/1 -> LatPosition1/2(P1)  "P1x"
  Bus Selector1/1 -> Selector6/1, Selector5/1, Selector7/1, Selector8/1  "<CtrlAryLat>"
  Selector5/1 -> LatPosition1/3(P2)  "P2x"
  Selector8/1 -> LatPosition1/1(P0)  "P0x"
  Selector7/1 -> LatPosition1/4(P3)  "P3x"
  Constant1/1 -> LatPosition1/5(tau)
  LatPosition1/1(y) -> Delay1/1
  Delay/1 -> Terminator/1  "PredHostPosnLgt"
  Delay1/1 -> Terminator1/1  "PredHostPosnLat"
  LatPosition1/2(dy) -> Terminator_1/1
  LongPosition1/2(dx) -> Terminator_2/1

== system TosAeb/AEB_Objsel/TA_Processor/AEB_ObjSelect/TargetInfoCheck/Data_precess/TA_EffectCalculate/Obj_FilterFlgCalculate/Long_ObjFilter/OverlapCalculate/BoudingBoxCalculate_Else/PosnatTTI/PredictObjectPositionAtTTR ==
  ttr : Inport
  Obj_Vx : Inport  Port=2
  Obj_Vy : Inport  Port=3
  Obj_Ax : Inport  Port=4
  Obj_Ay : Inport  Port=5
  Obj_dx : Inport  Port=6
  Obj_dy : Inport  Port=7
  TTCtemp : Inport  Port=8
  Add : Sum  Inputs=+++
  Add1 : Sum  Inputs=+++
  Add2 : Sum
  Add3 : Sum
  Compare To Constant : simulink/Logic and Bit Operations/Compare To Constant  relop=<=  const=0.2  OutDataTypeStr=boolean
  Compare To Constant1 : simulink/Logic and Bit Operations/Compare To Constant  relop=<=  const=4  OutDataTypeStr=boolean
  Constant : Constant  Value=0.5  OutDataTypeStr=single
  Constant1 : Constant  Value=0  OutDataTypeStr=single
  Constant3 : Constant  Value=4  OutDataTypeStr=single
  LP : Subsystem
  Product{,2..6} (6 alike) : Product
  Product1 : Product  Inputs=3
  Sin : Trigonometry  Operator=atan2
  Switch : Switch  Criteria=u2 ~= 0
  Switch1 : Switch  Criteria=u2 ~= 0
  Terminator : Terminator
  Terminator_1 : Terminator
  Obj_LongPosn : Outport
  Obj_LatPosn : Outport  Port=2
  Obj_PreHeading : Outport  Port=3
  wires:
  Obj_Vx/1 -> Product/2, [Obj_Vx]
  Constant/1 -> Product1/1
  Product1/1 -> [Facter]
  [Facter] -> Product2/1
  Obj_Ax/1 -> Product5/1, Product2/2
  Product/1 -> Add/1
  Add/1 -> Obj_LongPosn/1
  Obj_Vy/1 -> LP/1(Raw)
  [TTR] -> Product3/1
  [Facter] -> Product4/1
  Product3/1 -> Add1/1
  Add1/1 -> Obj_LatPosn/1
  Product2/1 -> Add/3
  Obj_dx/1 -> Add/2
  [TTR] -> Product5/2
  Product5/1 -> Add2/1
  [Obj_Vx] -> Add2/2
  Add2/1 -> [Obj_PreVx]
  Obj_dy/1 -> Add1/2
  [TTR] -> Product6/2
  Product6/1 -> Add3/1
  [Obj_Vy] -> Add3/2
  Add3/1 -> Sin/1
  [Obj_PreVx] -> Sin/2
  Sin/1 -> Obj_PreHeading/1
  Obj_Ay/1 -> Switch/3, Compare To Constant/1
  Switch/1 -> Product6/1, Product4/2
  Compare To Constant/1 -> Switch/2
  Constant1/1 -> Switch/1
  LP/1(ADS_EngTorqReq) -> [Obj_Vy], Product3/2
  [TTR] -> Product1/2, Product1/3
  Compare To Constant1/1 -> Switch1/1, Switch1/2
  Switch1/1 -> [TTR]
  Constant3/1 -> Switch1/3
  [TTR] -> Product/1
  Product4/1 -> Add1/3
  ttr/1 -> Compare To Constant1/1, Terminator/1
  TTCtemp/1 -> Terminator_1/1

== system TosAeb/AEB_Objsel/TA_Processor/AEB_ObjSelect/TargetInfoCheck/Data_precess/TA_EffectCalculate/Obj_FilterFlgCalculate/Long_ObjFilter/OverlapCalculate/BoudingBoxCalculate_Else/PosnatTTI/PredictRelativePositionAtTTR ==
  Ref_Vx : Inport
  Ref_Vy : Inport  Port=2
  Ref_ax : Inport  Port=3
  Ref_ay : Inport  Port=4
  Obj_dx : Inport  Port=5
  Obj_dy : Inport  Port=6
  ttr : Inport  Port=7
  VehSelfData : Inport  Port=8
  Abs : Abs
  Add : Sum  Inputs=+++
  Add1 : Sum  Inputs=+++
  Bus Selector : BusSelector  OutputSignals=VehSelf.AEB_Intervention_ESP,VehSelf.ABS_VehicleSpeed
  Compare To Constant : simulink/Logic and Bit Operations/Compare To Constant  relop=>  const=0  OutDataTypeStr=boolean
  Compare To Constant1 : simulink/Logic and Bit Operations/Compare To Constant  relop=>=  const=4  OutDataTypeStr=boolean
  Compare To Constant2 : simulink/Logic and Bit Operations/Compare To Constant  relop=>=  const=0.2  OutDataTypeStr=boolean
  Constant : Constant  Value=0.5
  Constant3 : Constant  Value=0.5
  Constant4 : Constant  Value=4  OutDataTypeStr=single
  Constant5 : Constant  Value=0  OutDataTypeStr=single
  Divide : Product  Inputs=*/
  Logical Operator : Logic  Operator=NOT  AllPortsSameDT=off  OutDataTypeStr=boolean
  Product{,2..4} (4 alike) : Product
  Product1 : Product  Inputs=3
  Product5 : Product  Inputs=3
  Switch2 : Switch  Criteria=u2 > Threshold
  Switch3 : Switch  Criteria=u2 ~= 0
  Switch4 : Switch  Criteria=u2 ~= 0
  TTCtemp : Outport
  Relative_LongPosn : Outport  Port=2
  Relative_LatPosn : Outport  Port=3
  wires:
  [TTR] -> Product/1
  Constant/1 -> Product1/3
  Product1/1 -> [Flag]
  [Flag] -> Product2/1
  Product/1 -> Add/1
  Product2/1 -> Add/2
  Obj_dx/1 -> [dx], Add/3
  Add/1 -> Relative_LongPosn/1
  [TTR_Lat] -> Product3/1
  Product3/1 -> Add1/1
  Add1/1 -> Relative_LatPosn/1  "distance"
  VehSelfData/1 -> Bus Selector/1
  Bus Selector/1 -> Compare To Constant/1  "<AEB_Intervention_ESP>"
  Compare To Constant/1 -> [AEB_State]
  Ref_ax/1 -> Product2/2
  Product5/1 -> [LatFlag]
  Constant3/1 -> Product5/3
  Switch2/1 -> [TTR], Product1/2, Product1/1
  Compare To Constant1/1 -> Switch2/2
  Constant4/1 -> Switch2/1
  Ref_Vy/1 -> Product3/2
  Ref_Vx/1 -> Product/2
  Bus Selector/2 -> [Veh_LongSpd_mps]  "<ABS_VehicleSpeed>"
  [dx] -> Divide/1
  [Veh_LongSpd_mps] -> Divide/2
  Obj_dy/1 -> Add1/3  "dy"
  Product4/1 -> Add1/2
  [AEB_State] -> Logical Operator/1
  Logical Operator/1 -> Switch3/2
  Divide/1 -> [TTCtemp], Switch3/3
  Switch3/1 -> Product5/1, Product5/2, [TTR_Lat]
  [LatFlag] -> Product4/1
  Ref_ay/1 -> Abs/1
  Compare To Constant2/1 -> Switch4/2
  Constant5/1 -> Switch4/3
  Abs/1 -> Switch4/1, Compare To Constant2/1
  Switch4/1 -> Product4/2
  ttr/1 -> Switch2/3, Switch3/1, Compare To Constant1/1
  [TTCtemp] -> TTCtemp/1

== system TosAeb/AEB_Objsel/TA_Processor/AEB_ObjSelect/TargetInfoCheck/Data_precess/TA_EffectCalculate/Obj_FilterFlgCalculate/Long_ObjFilter/TTR_Calculate/CalculateTTRAndTTM_else/LongitudinalTTRComputer/CalculateTTI ==
  note: Use the normal setup if solution exists.
      | If no solution exists, try the alternative setup.
      | If no solution exists at all, output a default value for TTI.
  MotionDataNormalSetup : Inport
  MotionDataAlternativeSetup : Inport  Port=2
  EdgeDistanceNormalSetup : Inport  Port=3
  EdgeDistanceAlternativeSetup : Inport  Port=4
  VehSelfData : Inport  Port=5
  StoppingData : Inport  Port=6
  Bus Selector : BusSelector  OutputSignals=VehSelf.AEB_Intervention_ESP
  HostAndObjectMoving : Subsystem  TreatAsAtomicUnit=on
  StationaryHost : Subsystem  TreatAsAtomicUnit=on
  StationaryObject : Subsystem  TreatAsAtomicUnit=on
  MovingTTI : Outport
  StationaryHostTTI : Outport  Port=2
  StationaryObjectTTI : Outport  Port=3
  MovingSolutionExists : Outport  Port=4
  StationaryHostSolutionExists : Outport  Port=5
  StationaryObjectSolutionExists : Outport  Port=6
  wires:
  [EdgeSetup2] -> StationaryObject/4(EdgeDistanceAlternativeSetup)
  EdgeDistanceAlternativeSetup/1 -> [EdgeSetup2], StationaryHost/3(EdgeDistanceAlternativeSetup)
  [EdgeSetup1] -> StationaryObject/2(EdgeDistanceNormalSetup)
  EdgeDistanceNormalSetup/1 -> StationaryHost/1(EdgeDistanceNormalSetup), [EdgeSetup1]  "EdgeDistanceNormalSetup"
  [EdgeSetup2] -> HostAndObjectMoving/3(EdgeDistanceAlternativeSetup)
  [EdgeSetup1] -> HostAndObjectMoving/1(EdgeDistanceNormalSetup)
  [MotionSetup1] -> StationaryObject/3(MotionDataNormalSetup)
  [MotionSetup2] -> StationaryObject/5(MotionDataAlternativeSetup)
  MotionDataNormalSetup/1 -> [MotionSetup1], HostAndObjectMoving/2(MotionDataNormalSetup)  "MotionDataNormalSetup"
  MotionDataAlternativeSetup/1 -> [MotionSetup2], HostAndObjectMoving/4(MotionDataAlternativeSetup)  "MotionDataAlternativeSetup"
  StoppingData/1 -> StationaryObject/1(StoppingData), StationaryHost/6(StoppingData)
  [MotionSetup1] -> StationaryHost/2(MotionDataNormalSetup)
  [MotionSetup2] -> StationaryHost/4(MotionDataAlternativeSetup)
  HostAndObjectMoving/1(MovingTTI) -> MovingTTI/1
  StationaryHost/1(StationaryHostTTI) -> StationaryHostTTI/1
  StationaryObject/1(StationaryObjectTTI) -> StationaryObjectTTI/1
  HostAndObjectMoving/2(MovingSolutionExists) -> MovingSolutionExists/1
  StationaryHost/2(StationaryHostSolutionExists) -> StationaryHostSolutionExists/1
  StationaryObject/2(StationaryObjectSolutionExists) -> StationaryObjectSolutionExists/1
  VehSelfData/1 -> Bus Selector/1
  Bus Selector/1 -> [AEB_State], StationaryObject/6(AEB_State)  "<AEB_Intervention_ESP>"
  [AEB_State] -> HostAndObjectMoving/5(AEB_State)
  [AEB_State] -> StationaryHost/5(AEB_State)
